simulink model "simulation_environment_thesis"  (R2024b)
settings: Solver=VariableStepAuto  StartTime=0.0  StopTime=3200  RelTol=1e-3
values from neighbouring files (symbols this model uses):
  A = [1, dt; 0, 1]    [estimate_and_control_fully_actuated_with_current.m]
  A1 = [1, 0, 0, 0, dt, 0 ... (first row only)    [estimate_and_control_fully_actuated_with_current.m]
  alpha = 10    [estimate_and_control_fully_actuated_with_current.m]
  alpha_xy_1 = 1    [estimate_and_control_fully_actuated_with_current.m]
  alpha_z_1 = 5    [estimate_and_control_fully_actuated_with_current.m]
  Az1 = [1, dt ... (first row only)    [estimate_and_control_fully_actuated_with_current.m]
  B = [dt; 0]    [estimate_and_control_fully_actuated_with_current.m]
  B1 = [dt, 0 ... (first row only)    [estimate_and_control_fully_actuated_with_current.m]
  Bz1 = [dt ... (first row only)    [estimate_and_control_fully_actuated_with_current.m]
  C1 = [1, 0, 0, 0, 0, 0 ... (first row only)    [estimate_and_control_fully_actuated_with_current.m]
  circumference_x = min_dist_from_ds*cos(theta)    [estimate_and_control_fully_actuated_with_current.m]
  circumference_y = min_dist_from_ds*sin(theta)    [estimate_and_control_fully_actuated_with_current.m]
  const_current = [-r; -s; 0; 0]    [estimate_and_control_fully_actuated_with_current.m]
  csi_psi_1 = 0.0003    [estimate_and_control_fully_actuated_with_current.m]
  csi_r_1 = 0.0003    [estimate_and_control_fully_actuated_with_current.m]
  csi_vcx_1 = 0.0003    [estimate_and_control_fully_actuated_with_current.m]
  csi_vcy_1 = 0.0003    [estimate_and_control_fully_actuated_with_current.m]
  csi_vcz_1 = 0.005    [estimate_and_control_fully_actuated_with_current.m]
  csi_x_1 = 0.003    [estimate_and_control_fully_actuated_with_current.m]
  csi_y_1 = 0.003    [estimate_and_control_fully_actuated_with_current.m]
  csi_z_1 = 0.005    [estimate_and_control_fully_actuated_with_current.m]
  Cz1 = [1, 0]    [estimate_and_control_fully_actuated_with_current.m]
  d = 1    [estimate_and_control_fully_actuated_with_current.m]
  delta_FOV = pi/7    (= 0.448798950513)    [estimate_and_control_fully_actuated_with_current.m]
  desired_speed = speed_0    [estimate_and_control_fully_actuated_with_current.m]
  dt = 1/Tsim    (= 0.005)    [estimate_and_control_fully_actuated_with_current.m]
  eta1 = deg2rad(0.5)    [estimate_and_control_fully_actuated_with_current.m]
  eta2 = deg2rad(0.5)    [estimate_and_control_fully_actuated_with_current.m]
  eta_psi_1 = 0.003    [estimate_and_control_fully_actuated_with_current.m]
  eta_x_1 = 0.03    [estimate_and_control_fully_actuated_with_current.m]
  eta_y_1 = 0.03    [estimate_and_control_fully_actuated_with_current.m]
  eta_z_1 = 0.005    [estimate_and_control_fully_actuated_with_current.m]
  G = dt*eye(2)    [estimate_and_control_fully_actuated_with_current.m]
  G1 = dt*eye(6)    [estimate_and_control_fully_actuated_with_current.m]
  Gz1 = dt*eye(2)    [estimate_and_control_fully_actuated_with_current.m]
  left_reference = L_x*[cos(NED_psi_ds); sin(NED_psi_ds); 0] + L_y_l*[-sin(NED_psi_ds); cos(NED_psi_ds); 0]    [estimate_and_control_fully_actuated_with_current.m]
  max_dist_from_ship = 60    [estimate_and_control_fully_actuated_with_current.m]
  min_dist_from_ds = 45    [estimate_and_control_fully_actuated_with_current.m]
  mu = -5    [estimate_and_control_fully_actuated_with_current.m]
  NED_psi_ds = -pi/2    (= -1.57079632679)    [estimate_and_control_fully_actuated_with_current.m]
  p_b1 = [-90; 90; 5]    [estimate_and_control_fully_actuated_with_current.m]
  position_t_0 = [0; 0; 0; 0]    [estimate_and_control_fully_actuated_with_current.m]
  psi_P = linspace(0,2*pi,360)'    [estimate_and_control_fully_actuated_with_current.m]
  Q = [csi1_kf^2, 0 ... (first row only)    [estimate_and_control_fully_actuated_with_current.m]
  Q1 = [csi_x_1_kf^2, 0, 0, 0, 0, 0 ... (first row only)    [estimate_and_control_fully_actuated_with_current.m]
  Qz1 = [csi_z_1_kf^2, 0 ... (first row only)    [estimate_and_control_fully_actuated_with_current.m]
  R = [eta1_kf^2, 0 ... (first row only)    [estimate_and_control_fully_actuated_with_current.m]
  R1 = [eta_x_1_kf^2, 0, 0 ... (first row only)    [estimate_and_control_fully_actuated_with_current.m]
  right_reference = L_x*[cos(NED_psi_ds); sin(NED_psi_ds); 0] + L_y_r*[sin(NED_psi_ds); -cos(NED_psi_ds); 0]    [estimate_and_control_fully_actuated_with_current.m]
  Rz1 = eta_z_1_kf^2    (= 1e-06)    [estimate_and_control_fully_actuated_with_current.m]
  speed_0 = [0.1, 0.1, 0, 0]'    [estimate_and_control_fully_actuated_with_current.m]
  tau_heave_sat = 30    [estimate_and_control_fully_actuated_with_current.m]
  tau_surge_sat = 10    [estimate_and_control_fully_actuated_with_current.m]
  tau_sway_sat = 30    [estimate_and_control_fully_actuated_with_current.m]
  tau_yaw_sat = 0.1    [estimate_and_control_fully_actuated_with_current.m]
  var_vm_x = 0.001    [estimate_and_control_fully_actuated_with_current.m]
  var_vm_y = 0.001    [estimate_and_control_fully_actuated_with_current.m]
  var_vm_z = 0.001    [estimate_and_control_fully_actuated_with_current.m]
undefined symbols (not defined in the file or in its folder): A2, alpha2, B2, csi1_2, csi2_2, csi3_2, csi4_2, csi5_2, csi6_2, csi7_2, csi8_2, eta1_2, eta2_2, eta3_2, eta4_2, eta5_2, eta6_2, G2, Q2, R2
libraries: simulink x60, simulink_extras x5
(part 8 of 16)

== system simulation_environment_thesis/PHASE 3&4  /PHASE  4/CONTROLLER4 ==
  v_err : Inport
  psi_err : Inport  Port=2
  speed_0 : Inport  Port=3  PortDimensions=[4,1]
  z_err : Inport  Port=4
  ANTIWINDUP SURGE : Subsystem
  ANTIWINDUP SWAY : Subsystem
  ANTIWINDUP_HEAVE : Subsystem
  ANTIWINDUP_YAW : Subsystem
  Demux : Demux
  MATLAB Function3 : MATLAB Function  TreatAsAtomicUnit=on
  Mux : Mux
  tau : Outport
  wires:
  MATLAB Function3/12(K_i_psi) -> ANTIWINDUP_YAW/1(K1)
  ANTIWINDUP SURGE/1(tau_u) -> Mux/1
  ANTIWINDUP_HEAVE/1(tau_w) -> Mux/3
  ANTIWINDUP_YAW/1(tau_psi) -> Mux/4
  MATLAB Function3/1(K_i_u) -> ANTIWINDUP SURGE/2(K)
  MATLAB Function3/3(K_t_u) -> ANTIWINDUP SURGE/4(K_t_u)
  MATLAB Function3/2(K_p_u) -> ANTIWINDUP SURGE/3(K1)
  speed_0/1 -> MATLAB Function3/1(speed_0)
  Demux/1 -> ANTIWINDUP SURGE/1(in)
  MATLAB Function3/14(K_t_psi) -> ANTIWINDUP_YAW/3(K_t_psi)
  MATLAB Function3/11(K_p_psi) -> ANTIWINDUP_YAW/2(K2)
  ANTIWINDUP SWAY/1(tau_v) -> Mux/2
  v_err/1 -> Demux/1
  Mux/1 -> tau/1
  psi_err/1 -> ANTIWINDUP_YAW/4(in1)
  Demux/2 -> ANTIWINDUP SWAY/1(in)
  MATLAB Function3/4(K_i_v) -> ANTIWINDUP SWAY/2(K)
  MATLAB Function3/5(K_p_v) -> ANTIWINDUP SWAY/3(K1)
  MATLAB Function3/6(K_t_v) -> ANTIWINDUP SWAY/4(K_t_v)
  MATLAB Function3/13(K_d_psi) -> ANTIWINDUP_YAW/6(K)
  Demux/4 -> ANTIWINDUP_YAW/5(in)
  MATLAB Function3/7(K_i_w) -> ANTIWINDUP_HEAVE/1(K1)
  MATLAB Function3/8(K_p_w) -> ANTIWINDUP_HEAVE/2(K2)
  MATLAB Function3/9(K_d_w) -> ANTIWINDUP_HEAVE/6(K)
  MATLAB Function3/10(K_t_w) -> ANTIWINDUP_HEAVE/3(K_t_w)
  Demux/3 -> ANTIWINDUP_HEAVE/5(in)
  z_err/1 -> ANTIWINDUP_HEAVE/4(in1)

== system simulation_environment_thesis/PHASE 3&4  /PHASE  4/EKF INIT ==
  direct_to_4 : Inport
  Data Store Read : DataStoreRead  DataStoreName=x_hat_23_4  IndexOptions=Index vector (dialog)  Indices=1  OutputSizes=1
  Data Store Read1 : DataStoreRead  DataStoreName=x_hat_34_4  IndexOptions=Index vector (dialog)  Indices=1  OutputSizes=1
  Data Store Read2 : DataStoreRead  DataStoreName=P0_23_4  IndexOptions=Index vector (dialog)  Indices=1  OutputSizes=1
  Data Store Read3 : DataStoreRead  DataStoreName=P0_34_4  IndexOptions=Index vector (dialog)  Indices=1  OutputSizes=1
  Switch3 : Switch  Criteria=u2 > Threshold  SaturateOnIntegerOverflow=off  InputSameDT=off
  Switch4 : Switch  Criteria=u2 > Threshold  SaturateOnIntegerOverflow=off  InputSameDT=off
  Out1 : Outport
  Out2 : Outport  Port=2
  wires:
  Switch4/1 -> Out2/1
  Data Store Read/1 -> Switch3/1
  Data Store Read2/1 -> Switch4/1
  Switch3/1 -> Out1/1
  Data Store Read1/1 -> Switch3/3
  direct_to_4/1 -> Switch3/2, Switch4/2
  Data Store Read3/1 -> Switch4/3

== system simulation_environment_thesis/PHASE 3&4  /PHASE  4/REFERENCE GENERATOR ==
  note: robot frame
  note: ok
  NED_psi_dock : Inport
  eta : Inport  Port=2
  ds_p_auv : Inport  Port=3
  v_tot_des : Inport  Port=4
  v_c : Inport  Port=5
  Constant : Constant  Value=min_dist_from_ds
  Constant10 : Constant  Value=[0;0]  VectorParams1D=off
  Constant11 : Constant  Value=15
  Constant12 : Constant  Value=speed_0
  Constant4 : Constant  Value=6
  Constant6 : Constant  Value=15
  Constant8 : Constant  Value=circumference_x  VectorParams1D=off
  Constant9 : Constant  Value=circumference_y  VectorParams1D=off
  MATLAB Function10 : MATLAB Function  TreatAsAtomicUnit=on
  MATLAB Function13 : MATLAB Function  TreatAsAtomicUnit=on
  MATLAB Function14 : MATLAB Function  TreatAsAtomicUnit=on
  MATLAB Function3 : MATLAB Function  TreatAsAtomicUnit=on
  MATLAB Function4 : MATLAB Function  TreatAsAtomicUnit=on
  MATLAB Function7 : MATLAB Function  TreatAsAtomicUnit=on
  Memory1 : Memory
  Memory2 : Memory  InitialCondition=psi_P
  Scope{4..5,7..12} (8 alike) : Scope
  v_blend : Outport
  wires:
  MATLAB Function13/1(v_lin) -> Scope5/1, MATLAB Function7/1(v_line)
  Constant10/1 -> MATLAB Function10/2(p0)
  Memory1/1 -> MATLAB Function14/5(beta)
  NED_psi_dock/1 -> MATLAB Function4/1(NED_psi_dock), MATLAB Function3/3(psi_dock), MATLAB Function13/3(psi_dock)
  MATLAB Function10/2(d) -> Scope7/1
  v_c/1 -> MATLAB Function13/2(v_curr), MATLAB Function3/2(v_c)
  Constant6/1 -> MATLAB Function10/5(D)
  MATLAB Function14/2(flag) -> Scope10/1
  MATLAB Function3/1(speed_desired) -> Scope8/1, MATLAB Function7/2(v_circ)
  Constant4/1 -> MATLAB Function10/6(alpha)
  MATLAB Function10/1(w) -> Scope4/1, MATLAB Function7/3(w)
  v_tot_des/1 -> MATLAB Function13/5(v_tot_des), MATLAB Function3/1(v_tot_des)
  MATLAB Function14/4(i) -> Scope11/1
  Constant8/1 -> MATLAB Function14/2(circ_x)
  MATLAB Function14/1(direction) -> Memory1/1, MATLAB Function3/5(beta), Scope12/1
  eta/1 -> MATLAB Function14/1(eta)
  MATLAB Function7/1(v_blend) -> Scope9/1, v_blend/1
  MATLAB Function14/3(psi_P) -> Memory2/1
  ds_p_auv/1 -> MATLAB Function10/1(xy), MATLAB Function13/1(r)
  Constant9/1 -> MATLAB Function14/3(circ_y)
  Constant11/1 -> MATLAB Function10/4(d0)
  Constant12/1 -> MATLAB Function3/4(speed0), MATLAB Function13/4(speed_0)
  Memory2/1 -> MATLAB Function14/4("psi_P ")
  MATLAB Function4/1(t) -> MATLAB Function10/3(t)
  Constant/1 -> MATLAB Function13/6(radius)

== system simulation_environment_thesis/PHASE 3&4  /PHASE  4/dynamic + kinematic models1 ==
  note: body frame
  note: ds frame
  note: body frame
  tau : Inport
  ref speed : Inport  Port=2
  last_yaw__from_ship : Inport  Port=3
  yaw_est : Inport  Port=4
  ds_p_auv : Inport  Port=5
  Constant : Constant  Value=0
  Demux : Demux
  Integrator1 : Integrator  InitialConditionSource=external
  Integrator2 : Integrator  InitialConditionSource=external
  Integrator4 : Integrator  InitialConditionSource=external  InitialCondition=speed_0  UpperSaturationLimit=10^3  LowerSaturationLimit=-10^3
  Integrator5 : Integrator  InitialCondition=const_current
  MATLAB Function : MATLAB Function  TreatAsAtomicUnit=on
  MATLAB Function7 : MATLAB Function  TreatAsAtomicUnit=on
  MATLAB Function9 : MATLAB Function  TreatAsAtomicUnit=on
  Mux : Mux  Inputs=2
  Mux1 : Mux  Inputs=2
  Mux3 : Mux  Inputs=3
  Mux4 : Mux
  Random Number : RandomNumber  Variance=csi1_2^2  Seed=410872712  SampleTime=dt
  Random Number1 : RandomNumber  Variance=csi3_2^2  Seed=715901785  SampleTime=dt
  Random Number10 : RandomNumber  Variance=csi8_2^2  Seed=436668268  SampleTime=dt
  Random Number3 : RandomNumber  Variance=csi6_2^2  Seed=2000657347  SampleTime=dt
  Random Number4 : RandomNumber  Variance=csi4_2^2  Seed=2009625762  SampleTime=dt
  Random Number5 : RandomNumber  Variance=csi2_2^2  Seed=1914756321  SampleTime=dt
  Random Number8 : RandomNumber  Variance=csi5_2^2  Seed=712966708  SampleTime=dt
  Random Number9 : RandomNumber  Variance=csi7_2^2  Seed=1551147939  SampleTime=dt
  Selector1 : Selector  InputPortWidth=4  IndexOptions=Index vector (dialog)  Indices=[4]  OutputSizes=1
  Selector2 : Selector  InputPortWidth=4  IndexOptions=Index vector (dialog)  Indices=[1 2 3]  OutputSizes=1
  Selector3 : Selector  InputPortWidth=4  IndexOptions=Index vector (dialog)  Indices=[1 2 3]  OutputSizes=1
  Sum{,1..2,5} (4 alike) : Sum  Inputs=|++
  Sum4 : Sum  Inputs=|+-
  error speed : Outport
  v_tot ds : Outport  Port=2
  p : Outport  Port=3
  yaw : Outport  Port=4
  body speed : Outport  Port=5
  wires:
  ref speed/1 -> Integrator4/2, Sum4/1
  MATLAB Function9/1(eta_dot) -> Sum5/1, Demux/1
  tau/1 -> MATLAB Function7/2(tau)
  Sum4/1 -> error speed/1
  Sum5/1 -> Selector3/1, v_tot ds/1
  Integrator4/1 -> MATLAB Function9/2(speed), MATLAB Function7/1(speed), Sum4/2, body speed/1
  Integrator5/1 -> Sum5/2
  last_yaw__from_ship/1 -> Integrator1/2
  Integrator1/1 -> MATLAB Function/1("angle ")
  yaw_est/1 -> MATLAB Function9/1(yaw)
  MATLAB Function/1(angle) -> Mux/2, yaw/1
  ds_p_auv/1 -> Integrator2/2
  Random Number1/1 -> Mux3/3
  Mux3/1 -> Sum/2
  Sum/1 -> Integrator2/1
  Random Number5/1 -> Mux3/2
  Random Number/1 -> Mux3/1
  Integrator2/1 -> Mux/1
  Random Number10/1 -> Sum2/2
  Selector1/1 -> Sum2/1
  Selector2/1 -> Mux1/1
  Sum2/1 -> Mux1/2
  Mux1/1 -> Integrator4/1
  MATLAB Function7/1(speed_dot) -> Selector2/1, Selector1/1
  Random Number9/1 -> Sum1/2
  Sum1/1 -> Integrator1/1
  Demux/4 -> Sum1/1
  Random Number8/1 -> Mux4/2
  Random Number4/1 -> Mux4/1
  Mux4/1 -> Integrator5/1
  Random Number3/1 -> Mux4/3
  Selector3/1 -> Sum/1
  Mux/1 -> p/1
  Constant/1 -> Mux4/4

== system simulation_environment_thesis/PHASE 3&4  /PHASE  4/p, v_c, psi, r (3D) estimate, from range, v_c, psi, z measurement ==
  note: x_k_kprev
  note: x_knext_k
  note: ship frame
  note: v
  note: doppler measurement noise
  note: u_k = v_m_k
  p : Inport
  inert_vel : Inport  Port=2
  psi : Inport  Port=3
  x_0_hat : Inport  Port=4
  P0 : Inport  Port=5
  Compare To Constant : simulink/Logic and Bit Operations/Compare To Constant  relop=>  const=0  OutDataTypeStr=boolean
  Compare To Constant1 : simulink/Logic and Bit Operations/Compare To Constant  relop=>  const=0  OutDataTypeStr=boolean
  Constant10 : Constant  Value=B2
  Constant12 : Constant  Value=const_current(1:3,1)  VectorParams1D=off
  Constant13 : Constant  Value=R2
  Constant14 : Constant  Value=A2
  Constant15 : Constant  Value=Q2
  Constant16 : Constant  Value=G2
  Constant9 : Constant  Value=A2  VectorParams1D=off
  Derivative : Derivative
  MATLAB Function : MATLAB Function  TreatAsAtomicUnit=on
  MATLAB Function10 : MATLAB Function  TreatAsAtomicUnit=on
  MATLAB Function6 : MATLAB Function  TreatAsAtomicUnit=on
  MATLAB Function7 : MATLAB Function  TreatAsAtomicUnit=on
  MATLAB Function8 : MATLAB Function  TreatAsAtomicUnit=on
  MATLAB Function9 : MATLAB Function  TreatAsAtomicUnit=on
  Memory1 : Memory
  Memory2 : Memory
  Mux : Mux  Inputs=6
  Mux1 : Mux
  Mux3 : Mux  Inputs=3
  Ramp : simulink/Sources/Ramp  slope=1  start=0  InitialOutput=0
  Ramp1 : simulink/Sources/Ramp  slope=1  start=0  InitialOutput=0
  Random Number1 : RandomNumber  Variance=eta1_2^2  Seed=222028095  SampleTime=dt
  Random Number11 : RandomNumber  Variance=eta3_2^2  Seed=796116377  SampleTime=dt
  Random Number12 : RandomNumber  Variance=eta5_2^2  Seed=1911211691  SampleTime=dt
  Random Number15 : RandomNumber  Variance=var_vm_z^2  Seed=217940665  SampleTime=dt
  Random Number16 : RandomNumber  Variance=eta6_2^2  Seed=1202889355  SampleTime=dt
  Random Number2 : RandomNumber  Variance=eta2_2^2  Seed=906863826  SampleTime=dt
  Random Number3 : RandomNumber  Variance=var_vm_x^2  Seed=1934193323  SampleTime=dt
  Random Number4 : RandomNumber  Variance=var_vm_y^2  Seed=128720054  SampleTime=dt
  Random Number8 : RandomNumber  Variance=eta4_2^2  Seed=177882395  SampleTime=dt
  Selector : Selector  InputPortWidth=3  IndexOptions=Index vector (dialog)  Indices=[3]  OutputSizes=1
  Selector1 : Selector  InputPortWidth=8  IndexOptions=Index vector (dialog)  Indices=[1 2 3]  OutputSizes=1
  Selector2 : Selector  InputPortWidth=4  IndexOptions=Index vector (dialog)  Indices=[1 2 3]  OutputSizes=1
  Selector3 : Selector  InputPortWidth=8  IndexOptions=Index vector (dialog)  Indices=[7]  OutputSizes=1
  Selector4 : Selector  InputPortWidth=8  IndexOptions=Index vector (dialog)  Indices=[4 5 6]  OutputSizes=1
  Selector6 : Selector  InputPortWidth=8  IndexOptions=Index vector (dialog)  Indices=[8]  OutputSizes=1
  Sum : Sum  Inputs=|++
  Sum5 : Sum  Inputs=|++
  Sum6 : Sum  Inputs=|+-
  Sum7 : Sum  Inputs=|++
  Sum8 : Sum  Inputs=|++-
  Switch : Switch  Criteria=u2 > Threshold  SaturateOnIntegerOverflow=off  InputSameDT=off
  Switch1 : Switch  Criteria=u2 > Threshold  SaturateOnIntegerOverflow=off  InputSameDT=off
  Unit Delay2 : UnitDelay  SampleTime=-1  HasFrameUpgradeWarning=on
  Unit Delay3 : UnitDelay  SampleTime=-1  HasFrameUpgradeWarning=on
  "psi " : Scope  NumInputPorts=2
  r : Scope  NumInputPorts=2
  v_curr_x_y_z : Scope  NumInputPorts=2
  x,y,z : Scope  NumInputPorts=2
  xyz_est : Outport
  psi_est : Outport  Port=2
  v_c_xyz : Outport  Port=3
  state_est : Outport  Port=4
  wires:
  MATLAB Function7/1(x_k_k) -> Sum5/2
  Constant14/1 -> MATLAB Function10/3(A)
  Switch/1 -> Selector3/1, Selector4/1, state_est/1, Selector6/1, Selector1/1, MATLAB Function10/6(x_k_kprev), Sum7/2, MATLAB Function6/1(x_k_kprev)
  Sum7/1 -> MATLAB Function7/2(u)
  Random Number4/1 -> Mux3/2
  MATLAB Function8/1(y) -> Sum5/1
  Random Number3/1 -> Mux3/1
  Constant15/1 -> MATLAB Function10/4(Q)
  MATLAB Function9/1(y) -> Sum7/1
  MATLAB Function10/2(P_knext_knext) -> Unit Delay3/1
  Sum8/1 -> MATLAB Function8/2(u)
  MATLAB Function10/1(K_knext) -> MATLAB Function9/1(K)
  p/1 -> Selector/1, x,y,z/2, MATLAB Function/1(p)
  Constant9/1 -> MATLAB Function7/1(A)
  Constant13/1 -> MATLAB Function10/2(R)
  Sum6/1 -> MATLAB Function9/2(y_err)
  Constant10/1 -> MATLAB Function8/1(B)
  Mux3/1 -> Sum8/1
  Constant16/1 -> MATLAB Function10/5(G)
  Sum5/1 -> Unit Delay2/1
  Sum/1 -> Sum6/1
  MATLAB Function6/1(y) -> Sum6/2
  Selector1/1 -> x,y,z/1, xyz_est/1
  psi/1 -> "psi "/2, Derivative/1, Mux1/3
  Mux1/1 -> Sum/1
  Selector3/1 -> "psi "/1, psi_est/1
  Selector4/1 -> v_curr_x_y_z/1, v_c_xyz/1
  inert_vel/1 -> Selector2/1
  Mux/1 -> Sum/2
  Random Number2/1 -> Mux/2
  Random Number11/1 -> Mux/3
  Random Number1/1 -> Mux/1
  MATLAB Function/1(y) -> Mux1/1
  Constant12/1 -> Mux1/2, Sum8/3, v_curr_x_y_z/2
  Random Number8/1 -> Mux/4
  Random Number12/1 -> Mux/5
  Derivative/1 -> r/1
  Selector6/1 -> r/2
  Random Number15/1 -> Mux3/3
  Selector2/1 -> Sum8/2
  Selector/1 -> Mux1/4
  Random Number16/1 -> Mux/6
  Ramp/1 -> Memory1/1
  Memory1/1 -> Compare To Constant/1
  Compare To Constant/1 -> Switch/2
  x_0_hat/1 -> Switch/3
  Unit Delay2/1 -> Switch/1
  Unit Delay3/1 -> Switch1/1
  Memory2/1 -> Compare To Constant1/1
  Compare To Constant1/1 -> Switch1/2
  Ramp1/1 -> Memory2/1
  P0/1 -> Switch1/3
  Switch1/1 -> MATLAB Function10/1(P_k_k)

== system simulation_environment_thesis/PHASE 3&4.21/PHASE  3/CONTROLLER4 ==
  v_err : Inport
  psi_err : Inport  Port=2
  speed_0 : Inport  Port=3
  z_err : Inport  Port=4
  ANTIWINDUP SURGE : Subsystem
  ANTIWINDUP SWAY : Subsystem
  ANTIWINDUP_HEAVE : Subsystem
  ANTIWINDUP_YAW : Subsystem
  Demux : Demux
  MATLAB Function3 : MATLAB Function  TreatAsAtomicUnit=on
  Mux : Mux
  tau : Outport
  wires:
  MATLAB Function3/12(K_i_psi) -> ANTIWINDUP_YAW/1(K1)
  ANTIWINDUP SURGE/1(tau_u) -> Mux/1
  ANTIWINDUP_HEAVE/1(tau_w) -> Mux/3
  ANTIWINDUP_YAW/1(tau_psi) -> Mux/4
  MATLAB Function3/1(K_i_u) -> ANTIWINDUP SURGE/2(K)
  MATLAB Function3/3(K_t_u) -> ANTIWINDUP SURGE/4(K_t_u)
  MATLAB Function3/2(K_p_u) -> ANTIWINDUP SURGE/3(K1)
  speed_0/1 -> MATLAB Function3/1(speed_0)
  Demux/1 -> ANTIWINDUP SURGE/1(in)
  MATLAB Function3/14(K_t_psi) -> ANTIWINDUP_YAW/3(K_t_psi)
  MATLAB Function3/11(K_p_psi) -> ANTIWINDUP_YAW/2(K2)
  ANTIWINDUP SWAY/1(tau_v) -> Mux/2
  v_err/1 -> Demux/1
  Mux/1 -> tau/1
  psi_err/1 -> ANTIWINDUP_YAW/4(in1)
  Demux/2 -> ANTIWINDUP SWAY/1(in)
  MATLAB Function3/4(K_i_v) -> ANTIWINDUP SWAY/2(K)
  MATLAB Function3/5(K_p_v) -> ANTIWINDUP SWAY/3(K1)
  MATLAB Function3/6(K_t_v) -> ANTIWINDUP SWAY/4(K_t_v)
  MATLAB Function3/13(K_d_psi) -> ANTIWINDUP_YAW/6(K)
  Demux/4 -> ANTIWINDUP_YAW/5(in)
  MATLAB Function3/7(K_i_w) -> ANTIWINDUP_HEAVE/1(K1)
  MATLAB Function3/8(K_p_w) -> ANTIWINDUP_HEAVE/2(K2)
  MATLAB Function3/9(K_d_w) -> ANTIWINDUP_HEAVE/6(K)
  MATLAB Function3/10(K_t_w) -> ANTIWINDUP_HEAVE/3(K_t_w)
  Demux/3 -> ANTIWINDUP_HEAVE/5(in)
  z_err/1 -> ANTIWINDUP_HEAVE/4(in1)

== system simulation_environment_thesis/PHASE 3&4.21/PHASE  3/XY PATH GUIDANCE ==
  note: nel sistema della DS, non della nave
  speed : Inport
  eta : Inport  Port=2
  yaw_est : Inport  Port=3
  v_c : Inport  Port=4
  Constant : Constant  Value=circumference_x  VectorParams1D=off
  Constant1 : Constant  Value=circumference_y  VectorParams1D=off
  Constant3 : Constant  Value=50
  Constant4 : Constant  Value=[0;0]  VectorParams1D=off
  Constant7 : Constant  Value=min_dist_from_ds
  MATLAB Function2 : MATLAB Function  TreatAsAtomicUnit=on
  MATLAB Function3 : MATLAB Function  TreatAsAtomicUnit=on
  MATLAB Function4 : MATLAB Function  TreatAsAtomicUnit=on
  MATLAB Function6 : MATLAB Function  TreatAsAtomicUnit=on
  MATLAB Function7 : MATLAB Function  TreatAsAtomicUnit=on
  Memory1 : Memory
  Memory2 : Memory  InitialCondition=psi_P
  Radians to Degrees : simulink_extras/Transformations/Radians to Degrees
  Scope : Scope
  Scope1 : Scope
  Scope2 : Scope
  desired yaw angle3 : Scope
  yaw_error : Outport
  wires:
  MATLAB Function3/1(psi) -> MATLAB Function7/2(alpha)
  MATLAB Function4/2(direction) -> Memory1/1, MATLAB Function3/1(psi_p), Radians to Degrees/1
  MATLAB Function4/1(y_1) -> MATLAB Function3/2(y_1)
  Memory2/1 -> MATLAB Function4/6("psi_P ")
  Constant7/1 -> MATLAB Function4/5(radius)
  Radians to Degrees/1 -> Scope2/1
  MATLAB Function4/3(flag) -> Scope/1
  Memory1/1 -> MATLAB Function4/7(prev_psi_P)
  MATLAB Function4/4(psi_P) -> Memory2/1
  Constant3/1 -> MATLAB Function3/3(delta_h)
  Constant4/1 -> MATLAB Function4/4(center)
  MATLAB Function2/1(yaw_des) -> desired yaw angle3/1, MATLAB Function6/1(yaw_ref)
  speed/1 -> MATLAB Function7/3(speed)
  Constant/1 -> MATLAB Function4/2(circ_x)
  Constant1/1 -> MATLAB Function4/3(circ_y)
  MATLAB Function7/1(yaw_desired) -> MATLAB Function2/1("yaw_des ")
  yaw_est/1 -> MATLAB Function6/2(yaw_est)
  MATLAB Function4/5(i) -> Scope1/1
  MATLAB Function6/1(yaw_error) -> yaw_error/1
  v_c/1 -> MATLAB Function7/1(v_c)
  eta/1 -> MATLAB Function4/1(eta)

== system simulation_environment_thesis/PHASE 3&4.21/PHASE  3/dynamic + kinematic models1 ==
  note: body frame
  note: ds frame
  note: body frame
  tau : Inport
  ref speed : Inport  Port=2
  last_yaw__from_ship : Inport  Port=3
  yaw_est : Inport  Port=4
  ds_p_auv : Inport  Port=5
  Constant : Constant  Value=0
  Demux : Demux
  Integrator1 : Integrator  InitialConditionSource=external
  Integrator2 : Integrator  InitialConditionSource=external
  Integrator4 : Integrator  InitialCondition=speed_0  UpperSaturationLimit=10^3  LowerSaturationLimit=-10^3
  Integrator5 : Integrator  InitialCondition=const_current
  MATLAB Function : MATLAB Function  TreatAsAtomicUnit=on
  MATLAB Function7 : MATLAB Function  TreatAsAtomicUnit=on
  MATLAB Function9 : MATLAB Function  TreatAsAtomicUnit=on
  Mux : Mux  Inputs=2
  Mux1 : Mux  Inputs=2
  Mux3 : Mux  Inputs=3
  Mux4 : Mux
  Random Number : RandomNumber  Variance=csi1_2^2  Seed=79473191  SampleTime=dt
  Random Number1 : RandomNumber  Variance=csi3_2^2  Seed=2010551213  SampleTime=dt
  Random Number10 : RandomNumber  Variance=csi8_2^2  Seed=1144351966  SampleTime=dt
  Random Number3 : RandomNumber  Variance=csi6_2^2  Seed=1475404124  SampleTime=dt
  Random Number4 : RandomNumber  Variance=csi4_2^2  Seed=1904706334  SampleTime=dt
  Random Number5 : RandomNumber  Variance=csi2_2^2  Seed=1410497516  SampleTime=dt
  Random Number8 : RandomNumber  Variance=csi5_2^2  Seed=470750039  SampleTime=dt
  Random Number9 : RandomNumber  Variance=csi7_2^2  Seed=1788756175  SampleTime=dt
  Selector1 : Selector  InputPortWidth=4  IndexOptions=Index vector (dialog)  Indices=[4]  OutputSizes=1
  Selector2 : Selector  InputPortWidth=4  IndexOptions=Index vector (dialog)  Indices=[1 2 3]  OutputSizes=1
  Selector3 : Selector  InputPortWidth=4  IndexOptions=Index vector (dialog)  Indices=[1 2 3]  OutputSizes=1
  Sum{,1..2,5} (4 alike) : Sum  Inputs=|++
  Sum4 : Sum  Inputs=|+-
  error speed : Outport
  v_tot ds : Outport  Port=2
  p : Outport  Port=3
  yaw : Outport  Port=4
  body speed : Outport  Port=5
  wires:
  ref speed/1 -> Sum4/1
  MATLAB Function9/1(eta_dot) -> Sum5/1, Demux/1
  tau/1 -> MATLAB Function7/2(tau)
  Sum4/1 -> error speed/1
  Sum5/1 -> Selector3/1, v_tot ds/1
  Integrator4/1 -> MATLAB Function7/1(speed), Sum4/2, body speed/1, MATLAB Function9/2(speed)
  Integrator5/1 -> Sum5/2
  last_yaw__from_ship/1 -> Integrator1/2
  Integrator1/1 -> MATLAB Function/1("angle ")
  yaw_est/1 -> MATLAB Function9/1(yaw)
  MATLAB Function/1(angle) -> Mux/2, yaw/1
  ds_p_auv/1 -> Integrator2/2
  Random Number1/1 -> Mux3/3
  Mux3/1 -> Sum/2
  Sum/1 -> Integrator2/1
  Random Number5/1 -> Mux3/2
  Random Number/1 -> Mux3/1
  Integrator2/1 -> Mux/1
  Random Number10/1 -> Sum2/2
  Selector1/1 -> Sum2/1
  Selector2/1 -> Mux1/1
  Sum2/1 -> Mux1/2
  Mux1/1 -> Integrator4/1
  MATLAB Function7/1(speed_dot) -> Selector2/1, Selector1/1
  Random Number9/1 -> Sum1/2
  Sum1/1 -> Integrator1/1
  Demux/4 -> Sum1/1
  Random Number8/1 -> Mux4/2
  Random Number4/1 -> Mux4/1
  Mux4/1 -> Integrator5/1
  Random Number3/1 -> Mux4/3
  Selector3/1 -> Sum/1
  Mux/1 -> p/1
  Constant/1 -> Mux4/4

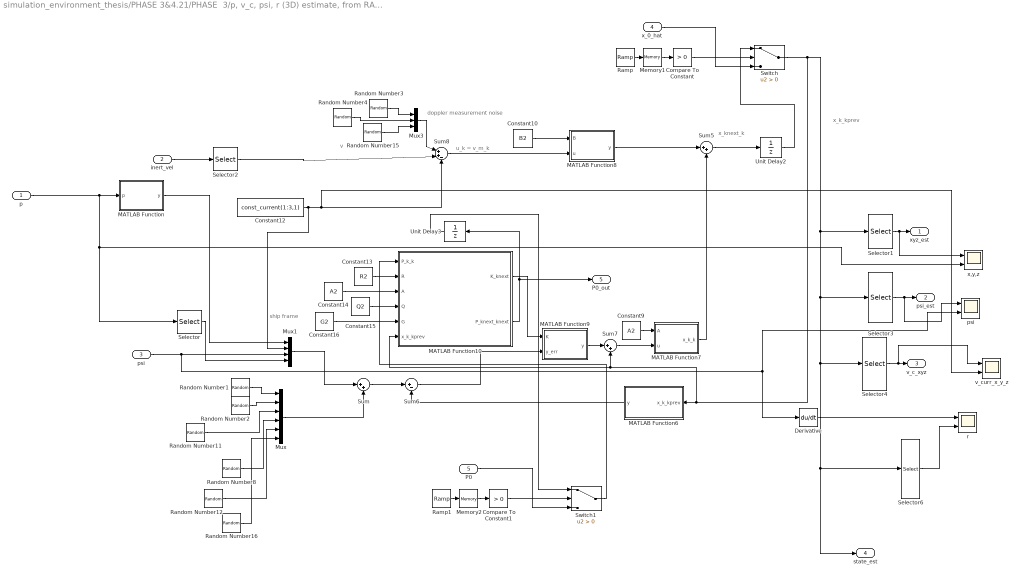
== system simulation_environment_thesis/PHASE 3&4.21/PHASE  3/p, v_c, psi, r (3D) estimate, from RANGE, v_c, PSI measurement ==
  note: x_k_kprev
  note: x_knext_k
  note: ship frame
  note: v
  note: doppler measurement noise
  note: u_k = v_m_k
  p : Inport
  inert_vel : Inport  Port=2
  psi : Inport  Port=3
  x_0_hat : Inport  Port=4
  P0 : Inport  Port=5
  Compare To Constant : simulink/Logic and Bit Operations/Compare To Constant  relop=>  const=0  OutDataTypeStr=boolean
  Compare To Constant1 : simulink/Logic and Bit Operations/Compare To Constant  relop=>  const=0  OutDataTypeStr=boolean
  Constant10 : Constant  Value=B2
  Constant12 : Constant  Value=const_current(1:3,1)  VectorParams1D=off
  Constant13 : Constant  Value=R2
  Constant14 : Constant  Value=A2
  Constant15 : Constant  Value=Q2
  Constant16 : Constant  Value=G2
  Constant9 : Constant  Value=A2  VectorParams1D=off
  Derivative : Derivative
  MATLAB Function : MATLAB Function  TreatAsAtomicUnit=on
  MATLAB Function10 : MATLAB Function  TreatAsAtomicUnit=on
  MATLAB Function6 : MATLAB Function  TreatAsAtomicUnit=on
  MATLAB Function7 : MATLAB Function  TreatAsAtomicUnit=on
  MATLAB Function8 : MATLAB Function  TreatAsAtomicUnit=on
  MATLAB Function9 : MATLAB Function  TreatAsAtomicUnit=on
  Memory1 : Memory
  Memory2 : Memory
  Mux : Mux  Inputs=6
  Mux1 : Mux
  Mux3 : Mux  Inputs=3
  Ramp : simulink/Sources/Ramp  slope=1  start=0  InitialOutput=0
  Ramp1 : simulink/Sources/Ramp  slope=1  start=0  InitialOutput=0
  Random Number1 : RandomNumber  Variance=eta1_2^2  Seed=726293252  SampleTime=dt
  Random Number11 : RandomNumber  Variance=eta3_2^2  Seed=1615333042  SampleTime=dt
  Random Number12 : RandomNumber  Variance=eta5_2^2  Seed=369043748  SampleTime=dt
  Random Number15 : RandomNumber  Variance=var_vm_z^2  Seed=734467476  SampleTime=dt
  Random Number16 : RandomNumber  Variance=eta6_2^2  Seed=866286033  SampleTime=dt
  Random Number2 : RandomNumber  Variance=eta2_2^2  Seed=150868444  SampleTime=dt
  Random Number3 : RandomNumber  Variance=var_vm_x^2  Seed=1760684058  SampleTime=dt
  Random Number4 : RandomNumber  Variance=var_vm_y^2  Seed=1048699801  SampleTime=dt
  Random Number8 : RandomNumber  Variance=eta4_2^2  Seed=301866519  SampleTime=dt
  Selector : Selector  InputPortWidth=3  IndexOptions=Index vector (dialog)  Indices=[3]  OutputSizes=1
  Selector1 : Selector  InputPortWidth=8  IndexOptions=Index vector (dialog)  Indices=[1 2 3]  OutputSizes=1
  Selector2 : Selector  InputPortWidth=4  IndexOptions=Index vector (dialog)  Indices=[1 2 3]  OutputSizes=1
  Selector3 : Selector  InputPortWidth=8  IndexOptions=Index vector (dialog)  Indices=[7]  OutputSizes=1
  Selector4 : Selector  InputPortWidth=8  IndexOptions=Index vector (dialog)  Indices=[4 5 6]  OutputSizes=1
  Selector6 : Selector  InputPortWidth=8  IndexOptions=Index vector (dialog)  Indices=[8]  OutputSizes=1
  Sum : Sum  Inputs=|++
  Sum5 : Sum  Inputs=|++
  Sum6 : Sum  Inputs=|+-
  Sum7 : Sum  Inputs=|++
  Sum8 : Sum  Inputs=|++-
  Switch : Switch  Criteria=u2 > Threshold  SaturateOnIntegerOverflow=off  InputSameDT=off
  Switch1 : Switch  Criteria=u2 > Threshold  SaturateOnIntegerOverflow=off  InputSameDT=off
  Unit Delay2 : UnitDelay  SampleTime=-1  HasFrameUpgradeWarning=on
  Unit Delay3 : UnitDelay  InitialCondition=alpha2*eye(8)  SampleTime=-1  HasFrameUpgradeWarning=on
  "psi " : Scope  NumInputPorts=2
  r : Scope  NumInputPorts=2
  v_curr_x_y_z : Scope  NumInputPorts=2
  x,y,z : Scope  NumInputPorts=2
  xyz_est : Outport
  psi_est : Outport  Port=2
  v_c_xyz : Outport  Port=3
  state_est : Outport  Port=4
  P0_out : Outport  Port=5
  wires:
  MATLAB Function7/1(x_k_k) -> Sum5/2
  Constant14/1 -> MATLAB Function10/3(A)
  Sum7/1 -> MATLAB Function7/2(u)
  Random Number4/1 -> Mux3/2
  MATLAB Function8/1(y) -> Sum5/1
  Random Number3/1 -> Mux3/1
  Constant15/1 -> MATLAB Function10/4(Q)
  MATLAB Function9/1(y) -> Sum7/1
  Sum5/1 -> Unit Delay2/1
  MATLAB Function10/2(P_knext_knext) -> P0_out/1, Unit Delay3/1
  Sum8/1 -> MATLAB Function8/2(u)
  MATLAB Function10/1(K_knext) -> MATLAB Function9/1(K)
  p/1 -> Selector/1, x,y,z/2, MATLAB Function/1(p)
  Constant9/1 -> MATLAB Function7/1(A)
  Constant13/1 -> MATLAB Function10/2(R)
  Sum6/1 -> MATLAB Function9/2(y_err)
  Constant10/1 -> MATLAB Function8/1(B)
  Mux3/1 -> Sum8/1
  Constant16/1 -> MATLAB Function10/5(G)
  Sum/1 -> Sum6/1
  MATLAB Function6/1(y) -> Sum6/2
  Selector1/1 -> x,y,z/1, xyz_est/1
  psi/1 -> "psi "/2, Derivative/1, Mux1/3
  Mux1/1 -> Sum/1
  Selector3/1 -> "psi "/1, psi_est/1
  Selector4/1 -> v_curr_x_y_z/1, v_c_xyz/1
  inert_vel/1 -> Selector2/1
  Mux/1 -> Sum/2
  Random Number2/1 -> Mux/2
  Random Number11/1 -> Mux/3
  Random Number1/1 -> Mux/1
  MATLAB Function/1(y) -> Mux1/1
  Constant12/1 -> Mux1/2, Sum8/3, v_curr_x_y_z/2
  Random Number8/1 -> Mux/4
  Random Number12/1 -> Mux/5
  Derivative/1 -> r/1
  Selector6/1 -> r/2
  Random Number15/1 -> Mux3/3
  Selector2/1 -> Sum8/2
  Random Number16/1 -> Mux/6
  Selector/1 -> Mux1/4
  Switch/1 -> Selector3/1, Selector4/1, state_est/1, Selector6/1, Selector1/1, MATLAB Function10/6(x_k_kprev), Sum7/2, MATLAB Function6/1(x_k_kprev)
  Ramp/1 -> Memory1/1
  Memory1/1 -> Compare To Constant/1
  Compare To Constant/1 -> Switch/2
  x_0_hat/1 -> Switch/3
  Unit Delay2/1 -> Switch/1
  Unit Delay3/1 -> Switch1/1
  Switch1/1 -> MATLAB Function10/1(P_k_k)
  Memory2/1 -> Compare To Constant1/1
  Compare To Constant1/1 -> Switch1/2
  Ramp1/1 -> Memory2/1
  P0/1 -> Switch1/3

== system simulation_environment_thesis/PHASE 3&4.21/PHASE  4/CONTROLLER4 ==
  v_err : Inport
  psi_err : Inport  Port=2
  speed_0 : Inport  Port=3  PortDimensions=[4,1]
  z_err : Inport  Port=4
  ANTIWINDUP SURGE : Subsystem
  ANTIWINDUP SWAY : Subsystem
  ANTIWINDUP_HEAVE : Subsystem
  ANTIWINDUP_YAW : Subsystem
  Demux : Demux
  MATLAB Function3 : MATLAB Function  TreatAsAtomicUnit=on
  Mux : Mux
  tau : Outport
  wires:
  MATLAB Function3/12(K_i_psi) -> ANTIWINDUP_YAW/1(K1)
  ANTIWINDUP SURGE/1(tau_u) -> Mux/1
  ANTIWINDUP_HEAVE/1(tau_w) -> Mux/3
  ANTIWINDUP_YAW/1(tau_psi) -> Mux/4
  MATLAB Function3/1(K_i_u) -> ANTIWINDUP SURGE/2(K)
  MATLAB Function3/3(K_t_u) -> ANTIWINDUP SURGE/4(K_t_u)
  MATLAB Function3/2(K_p_u) -> ANTIWINDUP SURGE/3(K1)
  speed_0/1 -> MATLAB Function3/1(speed_0)
  Demux/1 -> ANTIWINDUP SURGE/1(in)
  MATLAB Function3/14(K_t_psi) -> ANTIWINDUP_YAW/3(K_t_psi)
  MATLAB Function3/11(K_p_psi) -> ANTIWINDUP_YAW/2(K2)
  ANTIWINDUP SWAY/1(tau_v) -> Mux/2
  v_err/1 -> Demux/1
  Mux/1 -> tau/1
  psi_err/1 -> ANTIWINDUP_YAW/4(in1)
  Demux/2 -> ANTIWINDUP SWAY/1(in)
  MATLAB Function3/4(K_i_v) -> ANTIWINDUP SWAY/2(K)
  MATLAB Function3/5(K_p_v) -> ANTIWINDUP SWAY/3(K1)
  MATLAB Function3/6(K_t_v) -> ANTIWINDUP SWAY/4(K_t_v)
  MATLAB Function3/13(K_d_psi) -> ANTIWINDUP_YAW/6(K)
  Demux/4 -> ANTIWINDUP_YAW/5(in)
  MATLAB Function3/7(K_i_w) -> ANTIWINDUP_HEAVE/1(K1)
  MATLAB Function3/8(K_p_w) -> ANTIWINDUP_HEAVE/2(K2)
  MATLAB Function3/9(K_d_w) -> ANTIWINDUP_HEAVE/6(K)
  MATLAB Function3/10(K_t_w) -> ANTIWINDUP_HEAVE/3(K_t_w)
  Demux/3 -> ANTIWINDUP_HEAVE/5(in)
  z_err/1 -> ANTIWINDUP_HEAVE/4(in1)

== system simulation_environment_thesis/PHASE 3&4.21/PHASE  4/Subsystem ==
  note: ok
  note: robot frame
  NED_psi_dock : Inport
  x_y : Inport  Port=2
  eta : Inport  Port=3
  v_c : Inport  Port=4
  v_tot_des : Inport  Port=5
  Constant : Constant  Value=[0;0]  VectorParams1D=off
  Constant1 : Constant  Value=right_reference
  Constant10 : Constant  Value=min_dist_from_ds
  Constant2 : Constant  Value=left_reference
  Constant3 : Constant  Value=15
  Constant4 : Constant  Value=6
  Constant5 : Constant  Value=speed_0
  Constant6 : Constant  Value=15
  Constant8 : Constant  Value=circumference_x  VectorParams1D=off
  Constant9 : Constant  Value=circumference_y  VectorParams1D=off
  MATLAB Function1 : MATLAB Function  Commented=on  TreatAsAtomicUnit=on
  MATLAB Function10 : MATLAB Function  TreatAsAtomicUnit=on
  MATLAB Function13 : MATLAB Function  TreatAsAtomicUnit=on
  MATLAB Function2 : MATLAB Function  TreatAsAtomicUnit=on
  MATLAB Function3 : MATLAB Function  TreatAsAtomicUnit=on
  MATLAB Function4 : MATLAB Function  TreatAsAtomicUnit=on
  MATLAB Function7 : MATLAB Function  TreatAsAtomicUnit=on
  Memory1 : Memory
  Memory2 : Memory  InitialCondition=psi_P
  Scope{3..4,6..7,10..12} (7 alike) : Scope
  Scope2 : Scope  NumInputPorts=2
  Unit Delay2 : UnitDelay  SampleTime=-1  HasFrameUpgradeWarning=on
  w : Outport
  speed_desired_circ : Outport  Port=2
  d : Outport  Port=3
  speed_desired_lin : Outport  Port=4
  wires:
  MATLAB Function10/2(d) -> d/1, Scope7/1
  Constant6/1 -> MATLAB Function10/5(D)
  v_tot_des/1 -> MATLAB Function3/1(v_tot_des), MATLAB Function13/5(v_tot_des)
  MATLAB Function3/1(speed_desired) -> speed_desired_circ/1
  Constant5/1 -> MATLAB Function3/4(speed0), MATLAB Function13/4(speed_0)
  v_c/1 -> MATLAB Function3/2(v_c), MATLAB Function13/2(v_curr)
  Constant4/1 -> MATLAB Function10/6(alpha)
  Constant3/1 -> MATLAB Function10/4(d0)
  MATLAB Function4/1(t) -> MATLAB Function10/3(t)
  Constant/1 -> MATLAB Function10/2(p0)
  MATLAB Function10/1(w) -> w/1, Scope4/1
  Constant2/1 -> MATLAB Function2/2(left_trans)
  eta/1 -> MATLAB Function7/1(eta)
  NED_psi_dock/1 -> MATLAB Function4/1(NED_psi_dock), MATLAB Function3/3(psi_dock), MATLAB Function13/3(psi_dock)
  Memory2/1 -> MATLAB Function7/4("psi_P ")
  Unit Delay2/1 -> MATLAB Function2/4("stop_flag "), Scope3/1
  Memory1/1 -> MATLAB Function7/5(beta)
  x_y/1 -> MATLAB Function10/1(xy), MATLAB Function13/1(r), Scope6/1, MATLAB Function2/3(ds_p_auv)
  Constant9/1 -> MATLAB Function7/3(circ_y)
  MATLAB Function7/1(direction) -> Memory1/1, MATLAB Function3/5(beta), Scope12/1
  MATLAB Function7/3(psi_P) -> Memory2/1
  MATLAB Function2/3(P_L_range) -> Scope2/2
  MATLAB Function7/2(flag) -> Scope10/1
  MATLAB Function7/4(i) -> Scope11/1
  Constant1/1 -> MATLAB Function2/1(right_trans)
  MATLAB Function2/2(P_R_range) -> Scope2/1
  MATLAB Function2/1(stop_flag) -> Unit Delay2/1
  Constant8/1 -> MATLAB Function7/2(circ_x)
  Constant10/1 -> MATLAB Function13/6(radius)
  MATLAB Function13/1(v_lin) -> speed_desired_lin/1

== system simulation_environment_thesis/PHASE 3&4.21/PHASE  4/dynamic + kinematic models1 ==
  note: body frame
  note: ds frame
  note: body frame
  tau : Inport
  ref speed : Inport  Port=2
  last_yaw__from_ship : Inport  Port=3
  yaw_est : Inport  Port=4
  ds_p_auv : Inport  Port=5
  Constant : Constant  Value=0
  Demux : Demux
  Integrator1 : Integrator  InitialConditionSource=external
  Integrator2 : Integrator  InitialConditionSource=external
  Integrator4 : Integrator  InitialCondition=speed_0  UpperSaturationLimit=10^3  LowerSaturationLimit=-10^3
  Integrator5 : Integrator  InitialCondition=const_current
  MATLAB Function : MATLAB Function  TreatAsAtomicUnit=on
  MATLAB Function7 : MATLAB Function  TreatAsAtomicUnit=on
  MATLAB Function9 : MATLAB Function  TreatAsAtomicUnit=on
  Mux : Mux  Inputs=2
  Mux1 : Mux  Inputs=2
  Mux3 : Mux  Inputs=3
  Mux4 : Mux
  Random Number : RandomNumber  Variance=csi1_2^2  Seed=199152278  SampleTime=dt
  Random Number1 : RandomNumber  Variance=csi3_2^2  Seed=643503795  SampleTime=dt
  Random Number10 : RandomNumber  Variance=csi8_2^2  Seed=2081916765  SampleTime=dt
  Random Number3 : RandomNumber  Variance=csi6_2^2  Seed=713970042  SampleTime=dt
  Random Number4 : RandomNumber  Variance=csi4_2^2  Seed=168687267  SampleTime=dt
  Random Number5 : RandomNumber  Variance=csi2_2^2  Seed=656799255  SampleTime=dt
  Random Number8 : RandomNumber  Variance=csi5_2^2  Seed=2025524840  SampleTime=dt
  Random Number9 : RandomNumber  Variance=csi7_2^2  Seed=1667224033  SampleTime=dt
  Selector1 : Selector  InputPortWidth=4  IndexOptions=Index vector (dialog)  Indices=[4]  OutputSizes=1
  Selector2 : Selector  InputPortWidth=4  IndexOptions=Index vector (dialog)  Indices=[1 2 3]  OutputSizes=1
  Selector3 : Selector  InputPortWidth=4  IndexOptions=Index vector (dialog)  Indices=[1 2 3]  OutputSizes=1
  Sum{,1..2,5} (4 alike) : Sum  Inputs=|++
  Sum4 : Sum  Inputs=|+-
  error speed : Outport
  v_tot ds : Outport  Port=2
  p : Outport  Port=3
  yaw : Outport  Port=4
  body speed : Outport  Port=5
  wires:
  ref speed/1 -> Sum4/1
  MATLAB Function9/1(eta_dot) -> Sum5/1, Demux/1
  tau/1 -> MATLAB Function7/2(tau)
  Sum4/1 -> error speed/1
  Sum5/1 -> Selector3/1, v_tot ds/1
  Integrator4/1 -> MATLAB Function7/1(speed), Sum4/2, body speed/1, MATLAB Function9/2(speed)
  Integrator5/1 -> Sum5/2
  last_yaw__from_ship/1 -> Integrator1/2
  Integrator1/1 -> MATLAB Function/1("angle ")
  yaw_est/1 -> MATLAB Function9/1(yaw)
  MATLAB Function/1(angle) -> Mux/2, yaw/1
  ds_p_auv/1 -> Integrator2/2
  Random Number1/1 -> Mux3/3
  Mux3/1 -> Sum/2
  Sum/1 -> Integrator2/1
  Random Number5/1 -> Mux3/2
  Random Number/1 -> Mux3/1
  Integrator2/1 -> Mux/1
  Random Number10/1 -> Sum2/2
  Selector1/1 -> Sum2/1
  Selector2/1 -> Mux1/1
  Sum2/1 -> Mux1/2
  Mux1/1 -> Integrator4/1
  MATLAB Function7/1(speed_dot) -> Selector2/1, Selector1/1
  Random Number9/1 -> Sum1/2
  Sum1/1 -> Integrator1/1
  Demux/4 -> Sum1/1
  Random Number8/1 -> Mux4/2
  Random Number4/1 -> Mux4/1
  Mux4/1 -> Integrator5/1
  Random Number3/1 -> Mux4/3
  Selector3/1 -> Sum/1
  Mux/1 -> p/1
  Constant/1 -> Mux4/4

== system simulation_environment_thesis/PHASE 3&4.21/PHASE  4/p, v_c, psi, r (3D) estimate, from RANGE, v_c, PSI measurement ==
  note: x_k_kprev
  note: x_knext_k
  note: ship frame
  note: v
  note: doppler measurement noise
  note: u_k = v_m_k
  p : Inport
  inert_vel : Inport  Port=2
  psi : Inport  Port=3
  x_0_hat : Inport  Port=4
  P0 : Inport  Port=5
  Compare To Constant : simulink/Logic and Bit Operations/Compare To Constant  relop=>  const=0  OutDataTypeStr=boolean
  Compare To Constant1 : simulink/Logic and Bit Operations/Compare To Constant  relop=>  const=0  OutDataTypeStr=boolean
  Constant10 : Constant  Value=B2
  Constant12 : Constant  Value=const_current(1:3,1)  VectorParams1D=off
  Constant13 : Constant  Value=R2
  Constant14 : Constant  Value=A2
  Constant15 : Constant  Value=Q2
  Constant16 : Constant  Value=G2
  Constant9 : Constant  Value=A2  VectorParams1D=off
  Derivative : Derivative
  MATLAB Function : MATLAB Function  TreatAsAtomicUnit=on
  MATLAB Function10 : MATLAB Function  TreatAsAtomicUnit=on
  MATLAB Function6 : MATLAB Function  TreatAsAtomicUnit=on
  MATLAB Function7 : MATLAB Function  TreatAsAtomicUnit=on
  MATLAB Function8 : MATLAB Function  TreatAsAtomicUnit=on
  MATLAB Function9 : MATLAB Function  TreatAsAtomicUnit=on
  Memory1 : Memory
  Memory2 : Memory
  Mux : Mux  Inputs=6
  Mux1 : Mux
  Mux3 : Mux  Inputs=3
  Ramp : simulink/Sources/Ramp  slope=1  start=0  InitialOutput=0
  Ramp1 : simulink/Sources/Ramp  slope=1  start=0  InitialOutput=0
  Random Number1 : RandomNumber  Variance=eta1_2^2  Seed=60335897  SampleTime=dt
  Random Number11 : RandomNumber  Variance=eta3_2^2  Seed=1481451221  SampleTime=dt
  Random Number12 : RandomNumber  Variance=eta5_2^2  Seed=1773350443  SampleTime=dt
  Random Number15 : RandomNumber  Variance=var_vm_z^2  Seed=1051197454  SampleTime=dt
  Random Number16 : RandomNumber  Variance=eta6_2^2  Seed=422875429  SampleTime=dt
  Random Number2 : RandomNumber  Variance=eta2_2^2  Seed=172431280  SampleTime=dt
  Random Number3 : RandomNumber  Variance=var_vm_x^2  Seed=1322404624  SampleTime=dt
  Random Number4 : RandomNumber  Variance=var_vm_y^2  Seed=1266639097  SampleTime=dt
  Random Number8 : RandomNumber  Variance=eta4_2^2  Seed=1012276795  SampleTime=dt
  Selector : Selector  InputPortWidth=3  IndexOptions=Index vector (dialog)  Indices=[3]  OutputSizes=1
  Selector1 : Selector  InputPortWidth=8  IndexOptions=Index vector (dialog)  Indices=[1 2 3]  OutputSizes=1
  Selector2 : Selector  InputPortWidth=4  IndexOptions=Index vector (dialog)  Indices=[1 2 3]  OutputSizes=1
  Selector3 : Selector  InputPortWidth=8  IndexOptions=Index vector (dialog)  Indices=[7]  OutputSizes=1
  Selector4 : Selector  InputPortWidth=8  IndexOptions=Index vector (dialog)  Indices=[4 5 6]  OutputSizes=1
  Selector6 : Selector  InputPortWidth=8  IndexOptions=Index vector (dialog)  Indices=[8]  OutputSizes=1
  Sum : Sum  Inputs=|++
  Sum5 : Sum  Inputs=|++
  Sum6 : Sum  Inputs=|+-
  Sum7 : Sum  Inputs=|++
  Sum8 : Sum  Inputs=|++-
  Switch : Switch  Criteria=u2 > Threshold  SaturateOnIntegerOverflow=off  InputSameDT=off
  Switch1 : Switch  Criteria=u2 > Threshold  SaturateOnIntegerOverflow=off  InputSameDT=off
  Unit Delay2 : UnitDelay  SampleTime=-1  HasFrameUpgradeWarning=on
  Unit Delay3 : UnitDelay  InitialCondition=alpha2*eye(8)  SampleTime=-1  HasFrameUpgradeWarning=on
  "psi " : Scope  NumInputPorts=2
  r : Scope  NumInputPorts=2
  v_curr_x_y_z : Scope  NumInputPorts=2
  x,y,z : Scope  NumInputPorts=2
  xyz_est : Outport
  psi_est : Outport  Port=2
  v_c_xyz : Outport  Port=3
  state_est : Outport  Port=4
  wires:
  MATLAB Function7/1(x_k_k) -> Sum5/2
  Constant14/1 -> MATLAB Function10/3(A)
  Switch/1 -> Selector3/1, Selector4/1, state_est/1, Selector6/1, Selector1/1, MATLAB Function10/6(x_k_kprev), Sum7/2, MATLAB Function6/1(x_k_kprev)
  Sum7/1 -> MATLAB Function7/2(u)
  Random Number4/1 -> Mux3/2
  MATLAB Function8/1(y) -> Sum5/1
  Random Number3/1 -> Mux3/1
  Constant15/1 -> MATLAB Function10/4(Q)
  MATLAB Function9/1(y) -> Sum7/1
  MATLAB Function10/2(P_knext_knext) -> Unit Delay3/1
  Sum8/1 -> MATLAB Function8/2(u)
  MATLAB Function10/1(K_knext) -> MATLAB Function9/1(K)
  p/1 -> Selector/1, x,y,z/2, MATLAB Function/1(p)
  Constant9/1 -> MATLAB Function7/1(A)
  Constant13/1 -> MATLAB Function10/2(R)
  Sum6/1 -> MATLAB Function9/2(y_err)
  Constant10/1 -> MATLAB Function8/1(B)
  Mux3/1 -> Sum8/1
  Constant16/1 -> MATLAB Function10/5(G)
  Sum5/1 -> Unit Delay2/1
  Sum/1 -> Sum6/1
  MATLAB Function6/1(y) -> Sum6/2
  Selector1/1 -> x,y,z/1, xyz_est/1
  psi/1 -> "psi "/2, Derivative/1, Mux1/3
  Mux1/1 -> Sum/1
  Selector3/1 -> "psi "/1, psi_est/1
  Selector4/1 -> v_curr_x_y_z/1, v_c_xyz/1
  inert_vel/1 -> Selector2/1
  Mux/1 -> Sum/2
  Random Number2/1 -> Mux/2
  Random Number11/1 -> Mux/3
  Random Number1/1 -> Mux/1
  MATLAB Function/1(y) -> Mux1/1
  Constant12/1 -> Mux1/2, Sum8/3, v_curr_x_y_z/2
  Random Number8/1 -> Mux/4
  Random Number12/1 -> Mux/5
  Derivative/1 -> r/1
  Selector6/1 -> r/2
  Random Number15/1 -> Mux3/3
  Selector2/1 -> Sum8/2
  Selector/1 -> Mux1/4
  Random Number16/1 -> Mux/6
  Ramp/1 -> Memory1/1
  Memory1/1 -> Compare To Constant/1
  Compare To Constant/1 -> Switch/2
  x_0_hat/1 -> Switch/3
  Unit Delay2/1 -> Switch/1
  Unit Delay3/1 -> Switch1/1
  Memory2/1 -> Compare To Constant1/1
  Compare To Constant1/1 -> Switch1/2
  Ramp1/1 -> Memory2/1
  P0/1 -> Switch1/3
  Switch1/1 -> MATLAB Function10/1(P_k_k)

== system simulation_environment_thesis/PHASE 3&4.21/PHASE  4/positon and current estimate, XY PLANE from X, Y, psi measurement ==
  note: x_k_kprev
  note: x_knext_k
  note: ship frame
  note: [x
      | y]
  note: v
  note: doppler measurement noise
  note: u_k = v_m_k
  p : Inport
  inert_vel : Inport  Port=2
  psi : Inport  Port=3
  Constant : Constant  Value=C1
  Constant10 : Constant  Value=B1
  Constant12 : Constant  Value=const_current(1:2,1)  VectorParams1D=off
  Constant13 : Constant  Value=R1
  Constant14 : Constant  Value=A1
  Constant15 : Constant  Value=Q1
  Constant16 : Constant  Value=G1
  Constant9 : Constant  Value=A1  VectorParams1D=off
  Derivative : Derivative
  MATLAB Function10 : MATLAB Function  TreatAsAtomicUnit=on
  MATLAB Function6 : MATLAB Function  TreatAsAtomicUnit=on
  MATLAB Function7 : MATLAB Function  TreatAsAtomicUnit=on
  MATLAB Function8 : MATLAB Function  TreatAsAtomicUnit=on
  MATLAB Function9 : MATLAB Function  TreatAsAtomicUnit=on
  Mux : Mux  Inputs=3
  Mux1 : Mux  Inputs=2
  Mux3 : Mux  Inputs=2
  Mux4 : Mux  Inputs=6
  Random Number : RandomNumber  Variance=csi_x_1^2  SampleTime=dt
  Random Number1 : RandomNumber  Variance=eta_x_1^2  SampleTime=dt
  Random Number10 : RandomNumber  Variance=csi_r_1^2  SampleTime=dt
  Random Number13 : RandomNumber  Variance=eta_psi_1^2  SampleTime=dt
  Random Number2 : RandomNumber  Variance=eta_y_1^2  SampleTime=dt
  Random Number3 : RandomNumber  Variance=0.001  SampleTime=dt
  Random Number4 : RandomNumber  Variance=0.001  SampleTime=dt
  Random Number5 : RandomNumber  Variance=csi_y_1^2  SampleTime=dt
  Random Number6 : RandomNumber  Variance=csi_vcx_1^2  SampleTime=dt
  Random Number7 : RandomNumber  Variance=csi_vcy_1^2  SampleTime=dt
  Random Number9 : RandomNumber  Variance=csi_psi_1^2  SampleTime=dt
  Scope2 : Scope  NumInputPorts=5
  Selector : Selector  InputPortWidth=4  IndexOptions=Index vector (dialog)  Indices=[1 2]  OutputSizes=1
  Selector1 : Selector  InputPortWidth=6  IndexOptions=Index vector (dialog)  Indices=[1 2]  OutputSizes=1
  Selector2 : Selector  InputPortWidth=4  IndexOptions=Index vector (dialog)  Indices=[1 2]  OutputSizes=1
  Selector3 : Selector  InputPortWidth=6  IndexOptions=Index vector (dialog)  Indices=[3]  OutputSizes=1
  Selector4 : Selector  InputPortWidth=6  IndexOptions=Index vector (dialog)  Indices=[5 6]  OutputSizes=1
  Sum{,5,7,10} (4 alike) : Sum  Inputs=|++
  Sum6 : Sum  Inputs=|+-
  Sum8 : Sum  Inputs=|++-
  Unit Delay2 : UnitDelay  SampleTime=-1  HasFrameUpgradeWarning=on
  Unit Delay3 : UnitDelay  InitialCondition=alpha_xy_1*eye(6)  SampleTime=-1  HasFrameUpgradeWarning=on
  psi_est : Outport
  xy_est : Outport  Port=2
  Out3 : Outport  Port=3
  v_c_xy : Outport  Port=4
  wires:
  MATLAB Function7/1(x_k_k) -> Sum5/2
  Constant14/1 -> MATLAB Function10/3(A)
  Constant12/1 -> Sum8/3, Scope2/4
  Sum10/1 -> Selector3/1, Selector4/1, MATLAB Function6/1(x_k_kprev), Sum7/2, Selector1/1, Scope2/5
  Sum7/1 -> MATLAB Function7/2(u)
  Random Number4/1 -> Mux3/2
  MATLAB Function8/1(y) -> Sum5/1
  Random Number6/1 -> Mux4/5
  Random Number3/1 -> Mux3/1
  Random Number7/1 -> Mux4/6
  Constant15/1 -> MATLAB Function10/4(Q)
  MATLAB Function9/1(y) -> Sum7/1
  Unit Delay2/1 -> Sum10/1
  MATLAB Function10/2(P_knext_knext) -> Unit Delay3/1
  Sum8/1 -> MATLAB Function8/2(u)
  MATLAB Function10/1(K_knext) -> MATLAB Function9/1(K)
  Selector/1 -> Mux1/1, Scope2/1
  Constant9/1 -> MATLAB Function7/1(A)
  Constant13/1 -> MATLAB Function10/2(R)
  Sum6/1 -> MATLAB Function9/2(y_err)
  Constant10/1 -> MATLAB Function8/1(B)
  Unit Delay3/1 -> MATLAB Function10/1(P_k_k)
  Mux3/1 -> Sum8/1
  Random Number5/1 -> Mux4/2
  Mux4/1 -> Sum10/2
  Constant16/1 -> MATLAB Function10/5(G)
  Random Number/1 -> Mux4/1
  inert_vel/1 -> Selector2/1
  Sum5/1 -> Unit Delay2/1
  p/1 -> Selector/1
  Sum/1 -> Sum6/1
  MATLAB Function6/1(pred) -> Sum6/2
  Constant/1 -> MATLAB Function6/2(C), MATLAB Function10/6(C)
  Selector1/1 -> xy_est/1
  psi/1 -> Derivative/1, Scope2/2, Mux1/2
  Mux1/1 -> Sum/1
  Random Number9/1 -> Mux4/3
  Random Number10/1 -> Mux4/4
  Selector3/1 -> psi_est/1
  Selector4/1 -> v_c_xy/1
  Derivative/1 -> Scope2/3
  Selector2/1 -> Sum8/2
  Mux/1 -> Sum/2
  Random Number2/1 -> Mux/2
  Random Number13/1 -> Mux/3
  Random Number1/1 -> Mux/1
